simulink model "CCMVgaMotor"  (R2022b) (continued)
== system CCMVgaMotor == (continued)
  VentnActr04ElecErr_VentnActr04ElecErr/1 -> CCMVgaMotor_Runnable_sys/179(VentnActr04ElecErr_VentnActr04ElecErr_read)
  VentnActr04Mode_VentnActr04Mode/1 -> CCMVgaMotor_Runnable_sys/180(VentnActr04Mode_VentnActr04Mode_read)
  VentnActr04MovDir_VentnActr04MovDir/1 -> CCMVgaMotor_Runnable_sys/181(VentnActr04MovDir_VentnActr04MovDir_read)
  VentnActr04OvrTempErr_VentnActr04OvrTempErr/1 -> CCMVgaMotor_Runnable_sys/182(VentnActr04OvrTempErr_VentnActr04OvrTempErr_read)
  VentnActr04PsonSts_VentnActr04PsonSts/1 -> CCMVgaMotor_Runnable_sys/183(VentnActr04PsonSts_VentnActr04PsonSts_read)
  VentnActr04ResetSts_VentnActr04ResetSts/1 -> CCMVgaMotor_Runnable_sys/184(VentnActr04ResetSts_VentnActr04ResetSts_read)
  VentnActr04SpclFct_VentnActr04SpclFct/1 -> CCMVgaMotor_Runnable_sys/185(VentnActr04SpclFct_VentnActr04SpclFct_read)
  VentnActr04SpdSts_VentnActr04SpdSts/1 -> CCMVgaMotor_Runnable_sys/186(VentnActr04SpdSts_VentnActr04SpdSts_read)
  VentnActr04TrqSts_VentnActr04TrqSts/1 -> CCMVgaMotor_Runnable_sys/187(VentnActr04TrqSts_VentnActr04TrqSts_read)
  VentnActr04UrgcExist_VentnActr04UrgcExist/1 -> CCMVgaMotor_Runnable_sys/188(VentnActr04UrgcExist_VentnActr04UrgcExist_read)
  VentnActr04UrgcPson_VentnActr04UrgcPson/1 -> CCMVgaMotor_Runnable_sys/189(VentnActr04UrgcPson_VentnActr04UrgcPson_read)
  VentnActr04UrgcSts_VentnActr04UrgcSts/1 -> CCMVgaMotor_Runnable_sys/190(VentnActr04UrgcSts_VentnActr04UrgcSts_read)
  VentnActr04VoltErr_VentnActr04VoltErr/1 -> CCMVgaMotor_Runnable_sys/191(VentnActr04VoltErr_VentnActr04VoltErr_read)
  VentnActr05ActPson_VentnActr05ActPson/1 -> CCMVgaMotor_Runnable_sys/192(VentnActr05ActPson_VentnActr05ActPson_read)
  VentnActr05ActPson_VentnActr05ActPson_IsUpdated/1 -> CCMVgaMotor_Runnable_sys/193(VentnActr05ActPson_VentnActr05ActPson_IsUpdated_read)
  VentnActr05BlckInd_VentnActr05BlckInd/1 -> CCMVgaMotor_Runnable_sys/194(VentnActr05BlckInd_VentnActr05BlckInd_read)
  VentnActr05BlckSts_VentnActr05BlckSts/1 -> CCMVgaMotor_Runnable_sys/195(VentnActr05BlckSts_VentnActr05BlckSts_read)
  VentnActr05BusAdr_VentnActr05BusAdr/1 -> CCMVgaMotor_Runnable_sys/196(VentnActr05BusAdr_VentnActr05BusAdr_read)
  VentnActr05CoilSts_VentnActr05CoilSts/1 -> CCMVgaMotor_Runnable_sys/197(VentnActr05CoilSts_VentnActr05CoilSts_read)
  VentnActr05DrvDir_VentnActr05DrvDir/1 -> CCMVgaMotor_Runnable_sys/198(VentnActr05DrvDir_VentnActr05DrvDir_read)
  VentnActr05ElecErr_VentnActr05ElecErr/1 -> CCMVgaMotor_Runnable_sys/199(VentnActr05ElecErr_VentnActr05ElecErr_read)
  VentnActr05Mode_VentnActr05Mode/1 -> CCMVgaMotor_Runnable_sys/200(VentnActr05Mode_VentnActr05Mode_read)
  VentnActr05MovDir_VentnActr05MovDir/1 -> CCMVgaMotor_Runnable_sys/201(VentnActr05MovDir_VentnActr05MovDir_read)
  VentnActr05OvrTempErr_VentnActr05OvrTempErr/1 -> CCMVgaMotor_Runnable_sys/202(VentnActr05OvrTempErr_VentnActr05OvrTempErr_read)
  VentnActr05PsonSts_VentnActr05PsonSts/1 -> CCMVgaMotor_Runnable_sys/203(VentnActr05PsonSts_VentnActr05PsonSts_read)
  VentnActr05ResetSts_VentnActr05ResetSts/1 -> CCMVgaMotor_Runnable_sys/204(VentnActr05ResetSts_VentnActr05ResetSts_read)
  VentnActr05SpclFct_VentnActr05SpclFct/1 -> CCMVgaMotor_Runnable_sys/205(VentnActr05SpclFct_VentnActr05SpclFct_read)
  VentnActr05SpdSts_VentnActr05SpdSts/1 -> CCMVgaMotor_Runnable_sys/206(VentnActr05SpdSts_VentnActr05SpdSts_read)
  VentnActr05TrqSts_VentnActr05TrqSts/1 -> CCMVgaMotor_Runnable_sys/207(VentnActr05TrqSts_VentnActr05TrqSts_read)
  VentnActr05UrgcExist_VentnActr05UrgcExist/1 -> CCMVgaMotor_Runnable_sys/208(VentnActr05UrgcExist_VentnActr05UrgcExist_read)
  VentnActr05UrgcPson_VentnActr05UrgcPson/1 -> CCMVgaMotor_Runnable_sys/209(VentnActr05UrgcPson_VentnActr05UrgcPson_read)
  VentnActr05UrgcSts_VentnActr05UrgcSts/1 -> CCMVgaMotor_Runnable_sys/210(VentnActr05UrgcSts_VentnActr05UrgcSts_read)
  VentnActr05VoltErr_VentnActr05VoltErr/1 -> CCMVgaMotor_Runnable_sys/211(VentnActr05VoltErr_VentnActr05VoltErr_read)
  VentnActr06ActPson_VentnActr06ActPson/1 -> CCMVgaMotor_Runnable_sys/212(VentnActr06ActPson_VentnActr06ActPson_read)
  VentnActr06ActPson_VentnActr06ActPson_IsUpdated/1 -> CCMVgaMotor_Runnable_sys/213(VentnActr06ActPson_VentnActr06ActPson_IsUpdated_read)
  VentnActr06BlckInd_VentnActr06BlckInd/1 -> CCMVgaMotor_Runnable_sys/214(VentnActr06BlckInd_VentnActr06BlckInd_read)
  VentnActr06BlckSts_VentnActr06BlckSts/1 -> CCMVgaMotor_Runnable_sys/215(VentnActr06BlckSts_VentnActr06BlckSts_read)
  VentnActr06BusAdr_VentnActr06BusAdr/1 -> CCMVgaMotor_Runnable_sys/216(VentnActr06BusAdr_VentnActr06BusAdr_read)
  VentnActr06CoilSts_VentnActr06CoilSts/1 -> CCMVgaMotor_Runnable_sys/217(VentnActr06CoilSts_VentnActr06CoilSts_read)
  VentnActr06DrvDir_VentnActr06DrvDir/1 -> CCMVgaMotor_Runnable_sys/218(VentnActr06DrvDir_VentnActr06DrvDir_read)
  VentnActr06ElecErr_VentnActr06ElecErr/1 -> CCMVgaMotor_Runnable_sys/219(VentnActr06ElecErr_VentnActr06ElecErr_read)
  VentnActr06Mode_VentnActr06Mode/1 -> CCMVgaMotor_Runnable_sys/220(VentnActr06Mode_VentnActr06Mode_read)
  VentnActr06MovDir_VentnActr06MovDir/1 -> CCMVgaMotor_Runnable_sys/221(VentnActr06MovDir_VentnActr06MovDir_read)
  VentnActr06OvrTempErr_VentnActr06OvrTempErr/1 -> CCMVgaMotor_Runnable_sys/222(VentnActr06OvrTempErr_VentnActr06OvrTempErr_read)
  VentnActr06PsonSts_VentnActr06PsonSts/1 -> CCMVgaMotor_Runnable_sys/223(VentnActr06PsonSts_VentnActr06PsonSts_read)
  VentnActr06ResetSts_VentnActr06ResetSts/1 -> CCMVgaMotor_Runnable_sys/224(VentnActr06ResetSts_VentnActr06ResetSts_read)
  VentnActr06SpclFct_VentnActr06SpclFct/1 -> CCMVgaMotor_Runnable_sys/225(VentnActr06SpclFct_VentnActr06SpclFct_read)
  VentnActr06SpdSts_VentnActr06SpdSts/1 -> CCMVgaMotor_Runnable_sys/226(VentnActr06SpdSts_VentnActr06SpdSts_read)
  VentnActr06TrqSts_VentnActr06TrqSts/1 -> CCMVgaMotor_Runnable_sys/227(VentnActr06TrqSts_VentnActr06TrqSts_read)
  VentnActr06UrgcExist_VentnActr06UrgcExist/1 -> CCMVgaMotor_Runnable_sys/228(VentnActr06UrgcExist_VentnActr06UrgcExist_read)
  VentnActr06UrgcPson_VentnActr06UrgcPson/1 -> CCMVgaMotor_Runnable_sys/229(VentnActr06UrgcPson_VentnActr06UrgcPson_read)
  VentnActr06UrgcSts_VentnActr06UrgcSts/1 -> CCMVgaMotor_Runnable_sys/230(VentnActr06UrgcSts_VentnActr06UrgcSts_read)
  VentnActr06VoltErr_VentnActr06VoltErr/1 -> CCMVgaMotor_Runnable_sys/231(VentnActr06VoltErr_VentnActr06VoltErr_read)
  VentnActr07ActPson_VentnActr07ActPson/1 -> CCMVgaMotor_Runnable_sys/232(VentnActr07ActPson_VentnActr07ActPson_read)
  VentnActr07ActPson_VentnActr07ActPson_IsUpdated/1 -> CCMVgaMotor_Runnable_sys/233(VentnActr07ActPson_VentnActr07ActPson_IsUpdated_read)
  VentnActr07BlckInd_VentnActr07BlckInd/1 -> CCMVgaMotor_Runnable_sys/234(VentnActr07BlckInd_VentnActr07BlckInd_read)
  VentnActr07BlckSts_VentnActr07BlckSts/1 -> CCMVgaMotor_Runnable_sys/235(VentnActr07BlckSts_VentnActr07BlckSts_read)
  VentnActr07BusAdr_VentnActr07BusAdr/1 -> CCMVgaMotor_Runnable_sys/236(VentnActr07BusAdr_VentnActr07BusAdr_read)
  VentnActr07CoilSts_VentnActr07CoilSts/1 -> CCMVgaMotor_Runnable_sys/237(VentnActr07CoilSts_VentnActr07CoilSts_read)
  VentnActr07DrvDir_VentnActr07DrvDir/1 -> CCMVgaMotor_Runnable_sys/238(VentnActr07DrvDir_VentnActr07DrvDir_read)
  VentnActr07ElecErr_VentnActr07ElecErr/1 -> CCMVgaMotor_Runnable_sys/239(VentnActr07ElecErr_VentnActr07ElecErr_read)
  VentnActr07Mode_VentnActr07Mode/1 -> CCMVgaMotor_Runnable_sys/240(VentnActr07Mode_VentnActr07Mode_read)
  VentnActr07MovDir_VentnActr07MovDir/1 -> CCMVgaMotor_Runnable_sys/241(VentnActr07MovDir_VentnActr07MovDir_read)
  VentnActr07OvrTempErr_VentnActr07OvrTempErr/1 -> CCMVgaMotor_Runnable_sys/242(VentnActr07OvrTempErr_VentnActr07OvrTempErr_read)
  VentnActr07PsonSts_VentnActr07PsonSts/1 -> CCMVgaMotor_Runnable_sys/243(VentnActr07PsonSts_VentnActr07PsonSts_read)
  VentnActr07ResetSts_VentnActr07ResetSts/1 -> CCMVgaMotor_Runnable_sys/244(VentnActr07ResetSts_VentnActr07ResetSts_read)
  VentnActr07SpclFct_VentnActr07SpclFct/1 -> CCMVgaMotor_Runnable_sys/245(VentnActr07SpclFct_VentnActr07SpclFct_read)
  VentnActr07SpdSts_VentnActr07SpdSts/1 -> CCMVgaMotor_Runnable_sys/246(VentnActr07SpdSts_VentnActr07SpdSts_read)
  VentnActr07TrqSts_VentnActr07TrqSts/1 -> CCMVgaMotor_Runnable_sys/247(VentnActr07TrqSts_VentnActr07TrqSts_read)
  VentnActr07UrgcExist_VentnActr07UrgcExist/1 -> CCMVgaMotor_Runnable_sys/248(VentnActr07UrgcExist_VentnActr07UrgcExist_read)
  VentnActr07UrgcPson_VentnActr07UrgcPson/1 -> CCMVgaMotor_Runnable_sys/249(VentnActr07UrgcPson_VentnActr07UrgcPson_read)
  VentnActr07UrgcSts_VentnActr07UrgcSts/1 -> CCMVgaMotor_Runnable_sys/250(VentnActr07UrgcSts_VentnActr07UrgcSts_read)
  VentnActr07VoltErr_VentnActr07VoltErr/1 -> CCMVgaMotor_Runnable_sys/251(VentnActr07VoltErr_VentnActr07VoltErr_read)
  VentnActr08ActPson_VentnActr08ActPson/1 -> CCMVgaMotor_Runnable_sys/252(VentnActr08ActPson_VentnActr08ActPson_read)
  VentnActr08ActPson_VentnActr08ActPson_IsUpdated/1 -> CCMVgaMotor_Runnable_sys/253(VentnActr08ActPson_VentnActr08ActPson_IsUpdated_read)
  VentnActr08BlckInd_VentnActr08BlckInd/1 -> CCMVgaMotor_Runnable_sys/254(VentnActr08BlckInd_VentnActr08BlckInd_read)
  VentnActr08BlckSts_VentnActr08BlckSts/1 -> CCMVgaMotor_Runnable_sys/255(VentnActr08BlckSts_VentnActr08BlckSts_read)
  VentnActr08BusAdr_VentnActr08BusAdr/1 -> CCMVgaMotor_Runnable_sys/256(VentnActr08BusAdr_VentnActr08BusAdr_read)
  VentnActr08CoilSts_VentnActr08CoilSts/1 -> CCMVgaMotor_Runnable_sys/257(VentnActr08CoilSts_VentnActr08CoilSts_read)
  VentnActr08DrvDir_VentnActr08DrvDir/1 -> CCMVgaMotor_Runnable_sys/258(VentnActr08DrvDir_VentnActr08DrvDir_read)
  VentnActr08ElecErr_VentnActr08ElecErr/1 -> CCMVgaMotor_Runnable_sys/259(VentnActr08ElecErr_VentnActr08ElecErr_read)
  VentnActr08Mode_VentnActr08Mode/1 -> CCMVgaMotor_Runnable_sys/260(VentnActr08Mode_VentnActr08Mode_read)
  VentnActr08MovDir_VentnActr08MovDir/1 -> CCMVgaMotor_Runnable_sys/261(VentnActr08MovDir_VentnActr08MovDir_read)
  VentnActr08OvrTempErr_VentnActr08OvrTempErr/1 -> CCMVgaMotor_Runnable_sys/262(VentnActr08OvrTempErr_VentnActr08OvrTempErr_read)
  VentnActr08PsonSts_VentnActr08PsonSts/1 -> CCMVgaMotor_Runnable_sys/263(VentnActr08PsonSts_VentnActr08PsonSts_read)
  VentnActr08ResetSts_VentnActr08ResetSts/1 -> CCMVgaMotor_Runnable_sys/264(VentnActr08ResetSts_VentnActr08ResetSts_read)
  VentnActr08SpclFct_VentnActr08SpclFct/1 -> CCMVgaMotor_Runnable_sys/265(VentnActr08SpclFct_VentnActr08SpclFct_read)
  VentnActr08SpdSts_VentnActr08SpdSts/1 -> CCMVgaMotor_Runnable_sys/266(VentnActr08SpdSts_VentnActr08SpdSts_read)
  VentnActr08TrqSts_VentnActr08TrqSts/1 -> CCMVgaMotor_Runnable_sys/267(VentnActr08TrqSts_VentnActr08TrqSts_read)
  VentnActr08UrgcExist_VentnActr08UrgcExist/1 -> CCMVgaMotor_Runnable_sys/268(VentnActr08UrgcExist_VentnActr08UrgcExist_read)
  VentnActr08UrgcPson_VentnActr08UrgcPson/1 -> CCMVgaMotor_Runnable_sys/269(VentnActr08UrgcPson_VentnActr08UrgcPson_read)
  VentnActr08UrgcSts_VentnActr08UrgcSts/1 -> CCMVgaMotor_Runnable_sys/270(VentnActr08UrgcSts_VentnActr08UrgcSts_read)
  VentnActr08VoltErr_VentnActr08VoltErr/1 -> CCMVgaMotor_Runnable_sys/271(VentnActr08VoltErr_VentnActr08VoltErr_read)
  VentnActr09ActPson_VentnActr09ActPson/1 -> CCMVgaMotor_Runnable_sys/272(VentnActr09ActPson_VentnActr09ActPson_read)
  VentnActr09ActPson_VentnActr09ActPson_IsUpdated/1 -> CCMVgaMotor_Runnable_sys/273(VentnActr09ActPson_VentnActr09ActPson_IsUpdated_read)
  VentnActr09BlckInd_VentnActr09BlckInd/1 -> CCMVgaMotor_Runnable_sys/274(VentnActr09BlckInd_VentnActr09BlckInd_read)
  VentnActr09BlckSts_VentnActr09BlckSts/1 -> CCMVgaMotor_Runnable_sys/275(VentnActr09BlckSts_VentnActr09BlckSts_read)
  VentnActr09BusAdr_VentnActr09BusAdr/1 -> CCMVgaMotor_Runnable_sys/276(VentnActr09BusAdr_VentnActr09BusAdr_read)
  VentnActr09CoilSts_VentnActr09CoilSts/1 -> CCMVgaMotor_Runnable_sys/277(VentnActr09CoilSts_VentnActr09CoilSts_read)
  VentnActr09DrvDir_VentnActr09DrvDir/1 -> CCMVgaMotor_Runnable_sys/278(VentnActr09DrvDir_VentnActr09DrvDir_read)
  VentnActr09ElecErr_VentnActr09ElecErr/1 -> CCMVgaMotor_Runnable_sys/279(VentnActr09ElecErr_VentnActr09ElecErr_read)
  VentnActr09Mode_VentnActr09Mode/1 -> CCMVgaMotor_Runnable_sys/280(VentnActr09Mode_VentnActr09Mode_read)
  VentnActr09MovDir_VentnActr09MovDir/1 -> CCMVgaMotor_Runnable_sys/281(VentnActr09MovDir_VentnActr09MovDir_read)
  VentnActr09OvrTempErr_VentnActr09OvrTempErr/1 -> CCMVgaMotor_Runnable_sys/282(VentnActr09OvrTempErr_VentnActr09OvrTempErr_read)
  VentnActr09PsonSts_VentnActr09PsonSts/1 -> CCMVgaMotor_Runnable_sys/283(VentnActr09PsonSts_VentnActr09PsonSts_read)
  VentnActr09ResetSts_VentnActr09ResetSts/1 -> CCMVgaMotor_Runnable_sys/284(VentnActr09ResetSts_VentnActr09ResetSts_read)
  VentnActr09SpclFct_VentnActr09SpclFct/1 -> CCMVgaMotor_Runnable_sys/285(VentnActr09SpclFct_VentnActr09SpclFct_read)
  VentnActr09SpdSts_VentnActr09SpdSts/1 -> CCMVgaMotor_Runnable_sys/286(VentnActr09SpdSts_VentnActr09SpdSts_read)
  VentnActr09TrqSts_VentnActr09TrqSts/1 -> CCMVgaMotor_Runnable_sys/287(VentnActr09TrqSts_VentnActr09TrqSts_read)
  VentnActr09UrgcExist_VentnActr09UrgcExist/1 -> CCMVgaMotor_Runnable_sys/288(VentnActr09UrgcExist_VentnActr09UrgcExist_read)
  VentnActr09UrgcPson_VentnActr09UrgcPson/1 -> CCMVgaMotor_Runnable_sys/289(VentnActr09UrgcPson_VentnActr09UrgcPson_read)
  VentnActr09UrgcSts_VentnActr09UrgcSts/1 -> CCMVgaMotor_Runnable_sys/290(VentnActr09UrgcSts_VentnActr09UrgcSts_read)
  VentnActr09VoltErr_VentnActr09VoltErr/1 -> CCMVgaMotor_Runnable_sys/291(VentnActr09VoltErr_VentnActr09VoltErr_read)
  VentnActr10ActPson_VentnActr10ActPson/1 -> CCMVgaMotor_Runnable_sys/292(VentnActr10ActPson_VentnActr10ActPson_read)
  VentnActr10ActPson_VentnActr10ActPson_IsUpdated/1 -> CCMVgaMotor_Runnable_sys/293(VentnActr10ActPson_VentnActr10ActPson_IsUpdated_read)
  VentnActr10BlckInd_VentnActr10BlckInd/1 -> CCMVgaMotor_Runnable_sys/294(VentnActr10BlckInd_VentnActr10BlckInd_read)
  VentnActr10BlckSts_VentnActr10BlckSts/1 -> CCMVgaMotor_Runnable_sys/295(VentnActr10BlckSts_VentnActr10BlckSts_read)
  VentnActr10BusAdr_VentnActr10BusAdr/1 -> CCMVgaMotor_Runnable_sys/296(VentnActr10BusAdr_VentnActr10BusAdr_read)
  VentnActr10CoilSts_VentnActr10CoilSts/1 -> CCMVgaMotor_Runnable_sys/297(VentnActr10CoilSts_VentnActr10CoilSts_read)
  VentnActr10DrvDir_VentnActr10DrvDir/1 -> CCMVgaMotor_Runnable_sys/298(VentnActr10DrvDir_VentnActr10DrvDir_read)
  VentnActr10ElecErr_VentnActr10ElecErr/1 -> CCMVgaMotor_Runnable_sys/299(VentnActr10ElecErr_VentnActr10ElecErr_read)
  VentnActr10Mode_VentnActr10Mode/1 -> CCMVgaMotor_Runnable_sys/300(VentnActr10Mode_VentnActr10Mode_read)
  VentnActr10MovDir_VentnActr10MovDir/1 -> CCMVgaMotor_Runnable_sys/301(VentnActr10MovDir_VentnActr10MovDir_read)
  VentnActr10OvrTempErr_VentnActr10OvrTempErr/1 -> CCMVgaMotor_Runnable_sys/302(VentnActr10OvrTempErr_VentnActr10OvrTempErr_read)
  VentnActr10PsonSts_VentnActr10PsonSts/1 -> CCMVgaMotor_Runnable_sys/303(VentnActr10PsonSts_VentnActr10PsonSts_read)
  VentnActr10ResetSts_VentnActr10ResetSts/1 -> CCMVgaMotor_Runnable_sys/304(VentnActr10ResetSts_VentnActr10ResetSts_read)
  VentnActr10SpclFct_VentnActr10SpclFct/1 -> CCMVgaMotor_Runnable_sys/305(VentnActr10SpclFct_VentnActr10SpclFct_read)
  VentnActr10SpdSts_VentnActr10SpdSts/1 -> CCMVgaMotor_Runnable_sys/306(VentnActr10SpdSts_VentnActr10SpdSts_read)
  VentnActr10TrqSts_VentnActr10TrqSts/1 -> CCMVgaMotor_Runnable_sys/307(VentnActr10TrqSts_VentnActr10TrqSts_read)
  VentnActr10UrgcExist_VentnActr10UrgcExist/1 -> CCMVgaMotor_Runnable_sys/308(VentnActr10UrgcExist_VentnActr10UrgcExist_read)
  VentnActr10UrgcPson_VentnActr10UrgcPson/1 -> CCMVgaMotor_Runnable_sys/309(VentnActr10UrgcPson_VentnActr10UrgcPson_read)
  VentnActr10UrgcSts_VentnActr10UrgcSts/1 -> CCMVgaMotor_Runnable_sys/310(VentnActr10UrgcSts_VentnActr10UrgcSts_read)
  VentnActr10VoltErr_VentnActr10VoltErr/1 -> CCMVgaMotor_Runnable_sys/311(VentnActr10VoltErr_VentnActr10VoltErr_read)
  VentnActr11ActPson_VentnActr11ActPson/1 -> CCMVgaMotor_Runnable_sys/312(VentnActr11ActPson_VentnActr11ActPson_read)
  VentnActr11ActPson_VentnActr11ActPson_IsUpdated/1 -> CCMVgaMotor_Runnable_sys/313(VentnActr11ActPson_VentnActr11ActPson_IsUpdated_read)
  VentnActr11BlckInd_VentnActr11BlckInd/1 -> CCMVgaMotor_Runnable_sys/314(VentnActr11BlckInd_VentnActr11BlckInd_read)
  VentnActr11BlckSts_VentnActr11BlckSts/1 -> CCMVgaMotor_Runnable_sys/315(VentnActr11BlckSts_VentnActr11BlckSts_read)
  VentnActr11BusAdr_VentnActr11BusAdr/1 -> CCMVgaMotor_Runnable_sys/316(VentnActr11BusAdr_VentnActr11BusAdr_read)
  VentnActr11CoilSts_VentnActr11CoilSts/1 -> CCMVgaMotor_Runnable_sys/317(VentnActr11CoilSts_VentnActr11CoilSts_read)
  VentnActr11DrvDir_VentnActr11DrvDir/1 -> CCMVgaMotor_Runnable_sys/318(VentnActr11DrvDir_VentnActr11DrvDir_read)
  VentnActr11ElecErr_VentnActr11ElecErr/1 -> CCMVgaMotor_Runnable_sys/319(VentnActr11ElecErr_VentnActr11ElecErr_read)
  VentnActr11Mode_VentnActr11Mode/1 -> CCMVgaMotor_Runnable_sys/320(VentnActr11Mode_VentnActr11Mode_read)
  VentnActr11MovDir_VentnActr11MovDir/1 -> CCMVgaMotor_Runnable_sys/321(VentnActr11MovDir_VentnActr11MovDir_read)
  VentnActr11OvrTempErr_VentnActr11OvrTempErr/1 -> CCMVgaMotor_Runnable_sys/322(VentnActr11OvrTempErr_VentnActr11OvrTempErr_read)
  VentnActr11PsonSts_VentnActr11PsonSts/1 -> CCMVgaMotor_Runnable_sys/323(VentnActr11PsonSts_VentnActr11PsonSts_read)
  VentnActr11ResetSts_VentnActr11ResetSts/1 -> CCMVgaMotor_Runnable_sys/324(VentnActr11ResetSts_VentnActr11ResetSts_read)
  VentnActr11SpclFct_VentnActr11SpclFct/1 -> CCMVgaMotor_Runnable_sys/325(VentnActr11SpclFct_VentnActr11SpclFct_read)
  VentnActr11SpdSts_VentnActr11SpdSts/1 -> CCMVgaMotor_Runnable_sys/326(VentnActr11SpdSts_VentnActr11SpdSts_read)
  VentnActr11TrqSts_VentnActr11TrqSts/1 -> CCMVgaMotor_Runnable_sys/327(VentnActr11TrqSts_VentnActr11TrqSts_read)
  VentnActr11UrgcExist_VentnActr11UrgcExist/1 -> CCMVgaMotor_Runnable_sys/328(VentnActr11UrgcExist_VentnActr11UrgcExist_read)
  VentnActr11UrgcPson_VentnActr11UrgcPson/1 -> CCMVgaMotor_Runnable_sys/329(VentnActr11UrgcPson_VentnActr11UrgcPson_read)
  VentnActr11UrgcSts_VentnActr11UrgcSts/1 -> CCMVgaMotor_Runnable_sys/330(VentnActr11UrgcSts_VentnActr11UrgcSts_read)
  VentnActr11VoltErr_VentnActr11VoltErr/1 -> CCMVgaMotor_Runnable_sys/331(VentnActr11VoltErr_VentnActr11VoltErr_read)
  VentnActr12ActPson_VentnActr12ActPson/1 -> CCMVgaMotor_Runnable_sys/332(VentnActr12ActPson_VentnActr12ActPson_read)
  VentnActr12ActPson_VentnActr12ActPson_IsUpdated/1 -> CCMVgaMotor_Runnable_sys/333(VentnActr12ActPson_VentnActr12ActPson_IsUpdated_read)
  VentnActr12BlckInd_VentnActr12BlckInd/1 -> CCMVgaMotor_Runnable_sys/334(VentnActr12BlckInd_VentnActr12BlckInd_read)
  VentnActr12BlckSts_VentnActr12BlckSts/1 -> CCMVgaMotor_Runnable_sys/335(VentnActr12BlckSts_VentnActr12BlckSts_read)
  VentnActr12BusAdr_VentnActr12BusAdr/1 -> CCMVgaMotor_Runnable_sys/336(VentnActr12BusAdr_VentnActr12BusAdr_read)
  VentnActr12CoilSts_VentnActr12CoilSts/1 -> CCMVgaMotor_Runnable_sys/337(VentnActr12CoilSts_VentnActr12CoilSts_read)
  VentnActr12DrvDir_VentnActr12DrvDir/1 -> CCMVgaMotor_Runnable_sys/338(VentnActr12DrvDir_VentnActr12DrvDir_read)
  VentnActr12ElecErr_VentnActr12ElecErr/1 -> CCMVgaMotor_Runnable_sys/339(VentnActr12ElecErr_VentnActr12ElecErr_read)
  VentnActr12Mode_VentnActr12Mode/1 -> CCMVgaMotor_Runnable_sys/340(VentnActr12Mode_VentnActr12Mode_read)
  VentnActr12MovDir_VentnActr12MovDir/1 -> CCMVgaMotor_Runnable_sys/341(VentnActr12MovDir_VentnActr12MovDir_read)
  VentnActr12OvrTempErr_VentnActr12OvrTempErr/1 -> CCMVgaMotor_Runnable_sys/342(VentnActr12OvrTempErr_VentnActr12OvrTempErr_read)
  VentnActr12PsonSts_VentnActr12PsonSts/1 -> CCMVgaMotor_Runnable_sys/343(VentnActr12PsonSts_VentnActr12PsonSts_read)
  VentnActr12ResetSts_VentnActr12ResetSts/1 -> CCMVgaMotor_Runnable_sys/344(VentnActr12ResetSts_VentnActr12ResetSts_read)
  VentnActr12SpclFct_VentnActr12SpclFct/1 -> CCMVgaMotor_Runnable_sys/345(VentnActr12SpclFct_VentnActr12SpclFct_read)
  VentnActr12SpdSts_VentnActr12SpdSts/1 -> CCMVgaMotor_Runnable_sys/346(VentnActr12SpdSts_VentnActr12SpdSts_read)
  VentnActr12TrqSts_VentnActr12TrqSts/1 -> CCMVgaMotor_Runnable_sys/347(VentnActr12TrqSts_VentnActr12TrqSts_read)
  VentnActr12UrgcExist_VentnActr12UrgcExist/1 -> CCMVgaMotor_Runnable_sys/348(VentnActr12UrgcExist_VentnActr12UrgcExist_read)
  VentnActr12UrgcPson_VentnActr12UrgcPson/1 -> CCMVgaMotor_Runnable_sys/349(VentnActr12UrgcPson_VentnActr12UrgcPson_read)
  VentnActr12UrgcSts_VentnActr12UrgcSts/1 -> CCMVgaMotor_Runnable_sys/350(VentnActr12UrgcSts_VentnActr12UrgcSts_read)
  VentnActr12VoltErr_VentnActr12VoltErr/1 -> CCMVgaMotor_Runnable_sys/351(VentnActr12VoltErr_VentnActr12VoltErr_read)
  VentnActr13ActPson_VentnActr13ActPson/1 -> CCMVgaMotor_Runnable_sys/352(VentnActr13ActPson_VentnActr13ActPson_read)
  VentnActr13ActPson_VentnActr13ActPson_IsUpdated/1 -> CCMVgaMotor_Runnable_sys/353(VentnActr13ActPson_VentnActr13ActPson_IsUpdated_read)
  VentnActr13BlckInd_VentnActr13BlckInd/1 -> CCMVgaMotor_Runnable_sys/354(VentnActr13BlckInd_VentnActr13BlckInd_read)
  VentnActr13BlckSts_VentnActr13BlckSts/1 -> CCMVgaMotor_Runnable_sys/355(VentnActr13BlckSts_VentnActr13BlckSts_read)
  VentnActr13BusAdr_VentnActr13BusAdr/1 -> CCMVgaMotor_Runnable_sys/356(VentnActr13BusAdr_VentnActr13BusAdr_read)
  VentnActr13CoilSts_VentnActr13CoilSts/1 -> CCMVgaMotor_Runnable_sys/357(VentnActr13CoilSts_VentnActr13CoilSts_read)
  VentnActr13DrvDir_VentnActr13DrvDir/1 -> CCMVgaMotor_Runnable_sys/358(VentnActr13DrvDir_VentnActr13DrvDir_read)
  VentnActr13ElecErr_VentnActr13ElecErr/1 -> CCMVgaMotor_Runnable_sys/359(VentnActr13ElecErr_VentnActr13ElecErr_read)
  VentnActr13Mode_VentnActr13Mode/1 -> CCMVgaMotor_Runnable_sys/360(VentnActr13Mode_VentnActr13Mode_read)
  VentnActr13MovDir_VentnActr13MovDir/1 -> CCMVgaMotor_Runnable_sys/361(VentnActr13MovDir_VentnActr13MovDir_read)
  VentnActr13OvrTempErr_VentnActr13OvrTempErr/1 -> CCMVgaMotor_Runnable_sys/362(VentnActr13OvrTempErr_VentnActr13OvrTempErr_read)
  VentnActr13PsonSts_VentnActr13PsonSts/1 -> CCMVgaMotor_Runnable_sys/363(VentnActr13PsonSts_VentnActr13PsonSts_read)
  VentnActr13ResetSts_VentnActr13ResetSts/1 -> CCMVgaMotor_Runnable_sys/364(VentnActr13ResetSts_VentnActr13ResetSts_read)
  VentnActr13SpclFct_VentnActr13SpclFct/1 -> CCMVgaMotor_Runnable_sys/365(VentnActr13SpclFct_VentnActr13SpclFct_read)
  VentnActr13SpdSts_VentnActr13SpdSts/1 -> CCMVgaMotor_Runnable_sys/366(VentnActr13SpdSts_VentnActr13SpdSts_read)
  VentnActr13TrqSts_VentnActr13TrqSts/1 -> CCMVgaMotor_Runnable_sys/367(VentnActr13TrqSts_VentnActr13TrqSts_read)
  VentnActr13UrgcExist_VentnActr13UrgcExist/1 -> CCMVgaMotor_Runnable_sys/368(VentnActr13UrgcExist_VentnActr13UrgcExist_read)
  VentnActr13UrgcPson_VentnActr13UrgcPson/1 -> CCMVgaMotor_Runnable_sys/369(VentnActr13UrgcPson_VentnActr13UrgcPson_read)
  VentnActr13UrgcSts_VentnActr13UrgcSts/1 -> CCMVgaMotor_Runnable_sys/370(VentnActr13UrgcSts_VentnActr13UrgcSts_read)
  VentnActr13VoltErr_VentnActr13VoltErr/1 -> CCMVgaMotor_Runnable_sys/371(VentnActr13VoltErr_VentnActr13VoltErr_read)
  VentnActr14ActPson_VentnActr14ActPson/1 -> CCMVgaMotor_Runnable_sys/372(VentnActr14ActPson_VentnActr14ActPson_read)
  VentnActr14ActPson_VentnActr14ActPson_IsUpdated/1 -> CCMVgaMotor_Runnable_sys/373(VentnActr14ActPson_VentnActr14ActPson_IsUpdated_read)
  VentnActr14BlckInd_VentnActr14BlckInd/1 -> CCMVgaMotor_Runnable_sys/374(VentnActr14BlckInd_VentnActr14BlckInd_read)
  VentnActr14BlckSts_VentnActr14BlckSts/1 -> CCMVgaMotor_Runnable_sys/375(VentnActr14BlckSts_VentnActr14BlckSts_read)
  VentnActr14BusAdr_VentnActr14BusAdr/1 -> CCMVgaMotor_Runnable_sys/376(VentnActr14BusAdr_VentnActr14BusAdr_read)
  VentnActr14CoilSts_VentnActr14CoilSts/1 -> CCMVgaMotor_Runnable_sys/377(VentnActr14CoilSts_VentnActr14CoilSts_read)
  VentnActr14DrvDir_VentnActr14DrvDir/1 -> CCMVgaMotor_Runnable_sys/378(VentnActr14DrvDir_VentnActr14DrvDir_read)
  VentnActr14ElecErr_VentnActr14ElecErr/1 -> CCMVgaMotor_Runnable_sys/379(VentnActr14ElecErr_VentnActr14ElecErr_read)
  VentnActr14Mode_VentnActr14Mode/1 -> CCMVgaMotor_Runnable_sys/380(VentnActr14Mode_VentnActr14Mode_read)
  VentnActr14MovDir_VentnActr14MovDir/1 -> CCMVgaMotor_Runnable_sys/381(VentnActr14MovDir_VentnActr14MovDir_read)
  VentnActr14OvrTempErr_VentnActr14OvrTempErr/1 -> CCMVgaMotor_Runnable_sys/382(VentnActr14OvrTempErr_VentnActr14OvrTempErr_read)
  VentnActr14PsonSts_VentnActr14PsonSts/1 -> CCMVgaMotor_Runnable_sys/383(VentnActr14PsonSts_VentnActr14PsonSts_read)
  VentnActr14ResetSts_VentnActr14ResetSts/1 -> CCMVgaMotor_Runnable_sys/384(VentnActr14ResetSts_VentnActr14ResetSts_read)
  VentnActr14SpclFct_VentnActr14SpclFct/1 -> CCMVgaMotor_Runnable_sys/385(VentnActr14SpclFct_VentnActr14SpclFct_read)
  VentnActr14SpdSts_VentnActr14SpdSts/1 -> CCMVgaMotor_Runnable_sys/386(VentnActr14SpdSts_VentnActr14SpdSts_read)
  VentnActr14TrqSts_VentnActr14TrqSts/1 -> CCMVgaMotor_Runnable_sys/387(VentnActr14TrqSts_VentnActr14TrqSts_read)
  VentnActr14UrgcExist_VentnActr14UrgcExist/1 -> CCMVgaMotor_Runnable_sys/388(VentnActr14UrgcExist_VentnActr14UrgcExist_read)
  VentnActr14UrgcPson_VentnActr14UrgcPson/1 -> CCMVgaMotor_Runnable_sys/389(VentnActr14UrgcPson_VentnActr14UrgcPson_read)
  VentnActr14UrgcSts_VentnActr14UrgcSts/1 -> CCMVgaMotor_Runnable_sys/390(VentnActr14UrgcSts_VentnActr14UrgcSts_read)
  VentnActr14VoltErr_VentnActr14VoltErr/1 -> CCMVgaMotor_Runnable_sys/391(VentnActr14VoltErr_VentnActr14VoltErr_read)
  VentnActr15ActPson_VentnActr15ActPson/1 -> CCMVgaMotor_Runnable_sys/392(VentnActr15ActPson_VentnActr15ActPson_read)
  VentnActr15ActPson_VentnActr15ActPson_IsUpdated/1 -> CCMVgaMotor_Runnable_sys/393(VentnActr15ActPson_VentnActr15ActPson_IsUpdated_read)
  VentnActr15BlckInd_VentnActr15BlckInd/1 -> CCMVgaMotor_Runnable_sys/394(VentnActr15BlckInd_VentnActr15BlckInd_read)
  VentnActr15BlckSts_VentnActr15BlckSts/1 -> CCMVgaMotor_Runnable_sys/395(VentnActr15BlckSts_VentnActr15BlckSts_read)
  VentnActr15BusAdr_VentnActr15BusAdr/1 -> CCMVgaMotor_Runnable_sys/396(VentnActr15BusAdr_VentnActr15BusAdr_read)
  VentnActr15CoilSts_VentnActr15CoilSts/1 -> CCMVgaMotor_Runnable_sys/397(VentnActr15CoilSts_VentnActr15CoilSts_read)
  VentnActr15DrvDir_VentnActr15DrvDir/1 -> CCMVgaMotor_Runnable_sys/398(VentnActr15DrvDir_VentnActr15DrvDir_read)
  VentnActr15ElecErr_VentnActr15ElecErr/1 -> CCMVgaMotor_Runnable_sys/399(VentnActr15ElecErr_VentnActr15ElecErr_read)
  VentnActr15Mode_VentnActr15Mode/1 -> CCMVgaMotor_Runnable_sys/400(VentnActr15Mode_VentnActr15Mode_read)
  VentnActr15MovDir_VentnActr15MovDir/1 -> CCMVgaMotor_Runnable_sys/401(VentnActr15MovDir_VentnActr15MovDir_read)
  VentnActr15OvrTempErr_VentnActr15OvrTempErr/1 -> CCMVgaMotor_Runnable_sys/402(VentnActr15OvrTempErr_VentnActr15OvrTempErr_read)
  VentnActr15PsonSts_VentnActr15PsonSts/1 -> CCMVgaMotor_Runnable_sys/403(VentnActr15PsonSts_VentnActr15PsonSts_read)
  VentnActr15ResetSts_VentnActr15ResetSts/1 -> CCMVgaMotor_Runnable_sys/404(VentnActr15ResetSts_VentnActr15ResetSts_read)
  VentnActr15SpclFct_VentnActr15SpclFct/1 -> CCMVgaMotor_Runnable_sys/405(VentnActr15SpclFct_VentnActr15SpclFct_read)
  VentnActr15SpdSts_VentnActr15SpdSts/1 -> CCMVgaMotor_Runnable_sys/406(VentnActr15SpdSts_VentnActr15SpdSts_read)
  VentnActr15TrqSts_VentnActr15TrqSts/1 -> CCMVgaMotor_Runnable_sys/407(VentnActr15TrqSts_VentnActr15TrqSts_read)
  VentnActr15UrgcExist_VentnActr15UrgcExist/1 -> CCMVgaMotor_Runnable_sys/408(VentnActr15UrgcExist_VentnActr15UrgcExist_read)
  VentnActr15UrgcPson_VentnActr15UrgcPson/1 -> CCMVgaMotor_Runnable_sys/409(VentnActr15UrgcPson_VentnActr15UrgcPson_read)
  VentnActr15UrgcSts_VentnActr15UrgcSts/1 -> CCMVgaMotor_Runnable_sys/410(VentnActr15UrgcSts_VentnActr15UrgcSts_read)
  VentnActr15VoltErr_VentnActr15VoltErr/1 -> CCMVgaMotor_Runnable_sys/411(VentnActr15VoltErr_VentnActr15VoltErr_read)
  VentnActr16ActPson_VentnActr16ActPson/1 -> CCMVgaMotor_Runnable_sys/412(VentnActr16ActPson_VentnActr16ActPson_read)
  VentnActr16BlckInd_VentnActr16BlckInd/1 -> CCMVgaMotor_Runnable_sys/413(VentnActr16BlckInd_VentnActr16BlckInd_read)
  VentnActr16BlckSts_VentnActr16BlckSts/1 -> CCMVgaMotor_Runnable_sys/414(VentnActr16BlckSts_VentnActr16BlckSts_read)
  VentnActr16BusAdr_VentnActr16BusAdr/1 -> CCMVgaMotor_Runnable_sys/415(VentnActr16BusAdr_VentnActr16BusAdr_read)
  VentnActr16CoilSts_VentnActr16CoilSts/1 -> CCMVgaMotor_Runnable_sys/416(VentnActr16CoilSts_VentnActr16CoilSts_read)
  VentnActr16DrvDir_VentnActr16DrvDir/1 -> CCMVgaMotor_Runnable_sys/417(VentnActr16DrvDir_VentnActr16DrvDir_read)
  VentnActr16ElecErr_VentnActr16ElecErr/1 -> CCMVgaMotor_Runnable_sys/418(VentnActr16ElecErr_VentnActr16ElecErr_read)
  VentnActr16Mode_VentnActr16Mode/1 -> CCMVgaMotor_Runnable_sys/419(VentnActr16Mode_VentnActr16Mode_read)
  VentnActr16MovDir_VentnActr16MovDir/1 -> CCMVgaMotor_Runnable_sys/420(VentnActr16MovDir_VentnActr16MovDir_read)
  VentnActr16OvrTempErr_VentnActr16OvrTempErr/1 -> CCMVgaMotor_Runnable_sys/421(VentnActr16OvrTempErr_VentnActr16OvrTempErr_read)
  VentnActr16PsonSts_VentnActr16PsonSts/1 -> CCMVgaMotor_Runnable_sys/422(VentnActr16PsonSts_VentnActr16PsonSts_read)
  VentnActr16ResetSts_VentnActr16ResetSts/1 -> CCMVgaMotor_Runnable_sys/423(VentnActr16ResetSts_VentnActr16ResetSts_read)
  VentnActr16SpclFct_VentnActr16SpclFct/1 -> CCMVgaMotor_Runnable_sys/424(VentnActr16SpclFct_VentnActr16SpclFct_read)
  VentnActr16SpdSts_VentnActr16SpdSts/1 -> CCMVgaMotor_Runnable_sys/425(VentnActr16SpdSts_VentnActr16SpdSts_read)
  VentnActr16TrqSts_VentnActr16TrqSts/1 -> CCMVgaMotor_Runnable_sys/426(VentnActr16TrqSts_VentnActr16TrqSts_read)
  VentnActr16UrgcExist_VentnActr16UrgcExist/1 -> CCMVgaMotor_Runnable_sys/427(VentnActr16UrgcExist_VentnActr16UrgcExist_read)
  VentnActr16UrgcPson_VentnActr16UrgcPson/1 -> CCMVgaMotor_Runnable_sys/428(VentnActr16UrgcPson_VentnActr16UrgcPson_read)
  VentnActr16UrgcSts_VentnActr16UrgcSts/1 -> CCMVgaMotor_Runnable_sys/429(VentnActr16UrgcSts_VentnActr16UrgcSts_read)
  VentnActr16VoltErr_VentnActr16VoltErr/1 -> CCMVgaMotor_Runnable_sys/430(VentnActr16VoltErr_VentnActr16VoltErr_read)
  VentnActr17ActPson_VentnActr17ActPson/1 -> CCMVgaMotor_Runnable_sys/431(VentnActr17ActPson_VentnActr17ActPson_read)
  VentnActr17ActPson_VentnActr17ActPson_IsUpdated/1 -> CCMVgaMotor_Runnable_sys/432(VentnActr17ActPson_VentnActr17ActPson_IsUpdated_read)
  VentnActr17BlckInd_VentnActr17BlckInd/1 -> CCMVgaMotor_Runnable_sys/433(VentnActr17BlckInd_VentnActr17BlckInd_read)
  VentnActr17BlckSts_VentnActr17BlckSts/1 -> CCMVgaMotor_Runnable_sys/434(VentnActr17BlckSts_VentnActr17BlckSts_read)
  VentnActr17BusAdr_VentnActr17BusAdr/1 -> CCMVgaMotor_Runnable_sys/435(VentnActr17BusAdr_VentnActr17BusAdr_read)
  VentnActr17CoilSts_VentnActr17CoilSts/1 -> CCMVgaMotor_Runnable_sys/436(VentnActr17CoilSts_VentnActr17CoilSts_read)
  VentnActr17DrvDir_VentnActr17DrvDir/1 -> CCMVgaMotor_Runnable_sys/437(VentnActr17DrvDir_VentnActr17DrvDir_read)
  VentnActr17ElecErr_VentnActr17ElecErr/1 -> CCMVgaMotor_Runnable_sys/438(VentnActr17ElecErr_VentnActr17ElecErr_read)
  VentnActr17Mode_VentnActr17Mode/1 -> CCMVgaMotor_Runnable_sys/439(VentnActr17Mode_VentnActr17Mode_read)
  VentnActr17MovDir_VentnActr17MovDir/1 -> CCMVgaMotor_Runnable_sys/440(VentnActr17MovDir_VentnActr17MovDir_read)
  VentnActr17OvrTempErr_VentnActr17OvrTempErr/1 -> CCMVgaMotor_Runnable_sys/441(VentnActr17OvrTempErr_VentnActr17OvrTempErr_read)
  VentnActr17PsonSts_VentnActr17PsonSts/1 -> CCMVgaMotor_Runnable_sys/442(VentnActr17PsonSts_VentnActr17PsonSts_read)
  VentnActr17ResetSts_VentnActr17ResetSts/1 -> CCMVgaMotor_Runnable_sys/443(VentnActr17ResetSts_VentnActr17ResetSts_read)
  VentnActr17SpclFct_VentnActr17SpclFct/1 -> CCMVgaMotor_Runnable_sys/444(VentnActr17SpclFct_VentnActr17SpclFct_read)
  VentnActr17SpdSts_VentnActr17SpdSts/1 -> CCMVgaMotor_Runnable_sys/445(VentnActr17SpdSts_VentnActr17SpdSts_read)
  VentnActr17TrqSts_VentnActr17TrqSts/1 -> CCMVgaMotor_Runnable_sys/446(VentnActr17TrqSts_VentnActr17TrqSts_read)
  VentnActr17UrgcExist_VentnActr17UrgcExist/1 -> CCMVgaMotor_Runnable_sys/447(VentnActr17UrgcExist_VentnActr17UrgcExist_read)
  VentnActr17UrgcPson_VentnActr17UrgcPson/1 -> CCMVgaMotor_Runnable_sys/448(VentnActr17UrgcPson_VentnActr17UrgcPson_read)
  VentnActr17UrgcSts_VentnActr17UrgcSts/1 -> CCMVgaMotor_Runnable_sys/449(VentnActr17UrgcSts_VentnActr17UrgcSts_read)
  VentnActr17VoltErr_VentnActr17VoltErr/1 -> CCMVgaMotor_Runnable_sys/450(VentnActr17VoltErr_VentnActr17VoltErr_read)
  VentnActr18ActPson_VentnActr18ActPson/1 -> CCMVgaMotor_Runnable_sys/451(VentnActr18ActPson_VentnActr18ActPson_read)
  VentnActr18BlckInd_VentnActr18BlckInd/1 -> CCMVgaMotor_Runnable_sys/452(VentnActr18BlckInd_VentnActr18BlckInd_read)
  VentnActr18BlckSts_VentnActr18BlckSts/1 -> CCMVgaMotor_Runnable_sys/453(VentnActr18BlckSts_VentnActr18BlckSts_read)
  VentnActr18BusAdr_VentnActr18BusAdr/1 -> CCMVgaMotor_Runnable_sys/454(VentnActr18BusAdr_VentnActr18BusAdr_read)
  VentnActr18CoilSts_VentnActr18CoilSts/1 -> CCMVgaMotor_Runnable_sys/455(VentnActr18CoilSts_VentnActr18CoilSts_read)
  VentnActr18DrvDir_VentnActr18DrvDir/1 -> CCMVgaMotor_Runnable_sys/456(VentnActr18DrvDir_VentnActr18DrvDir_read)
  VentnActr18ElecErr_VentnActr18ElecErr/1 -> CCMVgaMotor_Runnable_sys/457(VentnActr18ElecErr_VentnActr18ElecErr_read)
  VentnActr18Mode_VentnActr18Mode/1 -> CCMVgaMotor_Runnable_sys/458(VentnActr18Mode_VentnActr18Mode_read)
  VentnActr18MovDir_VentnActr18MovDir/1 -> CCMVgaMotor_Runnable_sys/459(VentnActr18MovDir_VentnActr18MovDir_read)
  VentnActr18OvrTempErr_VentnActr18OvrTempErr/1 -> CCMVgaMotor_Runnable_sys/460(VentnActr18OvrTempErr_VentnActr18OvrTempErr_read)
  VentnActr18PsonSts_VentnActr18PsonSts/1 -> CCMVgaMotor_Runnable_sys/461(VentnActr18PsonSts_VentnActr18PsonSts_read)
  VentnActr18ResetSts_VentnActr18ResetSts/1 -> CCMVgaMotor_Runnable_sys/462(VentnActr18ResetSts_VentnActr18ResetSts_read)
  VentnActr18SpclFct_VentnActr18SpclFct/1 -> CCMVgaMotor_Runnable_sys/463(VentnActr18SpclFct_VentnActr18SpclFct_read)
  VentnActr18SpdSts_VentnActr18SpdSts/1 -> CCMVgaMotor_Runnable_sys/464(VentnActr18SpdSts_VentnActr18SpdSts_read)
  VentnActr18TrqSts_VentnActr18TrqSts/1 -> CCMVgaMotor_Runnable_sys/465(VentnActr18TrqSts_VentnActr18TrqSts_read)
  VentnActr18UrgcExist_VentnActr18UrgcExist/1 -> CCMVgaMotor_Runnable_sys/466(VentnActr18UrgcExist_VentnActr18UrgcExist_read)
  VentnActr18UrgcPson_VentnActr18UrgcPson/1 -> CCMVgaMotor_Runnable_sys/467(VentnActr18UrgcPson_VentnActr18UrgcPson_read)
  VentnActr18UrgcSts_VentnActr18UrgcSts/1 -> CCMVgaMotor_Runnable_sys/468(VentnActr18UrgcSts_VentnActr18UrgcSts_read)
  VentnActr18VoltErr_VentnActr18VoltErr/1 -> CCMVgaMotor_Runnable_sys/469(VentnActr18VoltErr_VentnActr18VoltErr_read)
  VentnActr19ActPson_VentnActr19ActPson/1 -> CCMVgaMotor_Runnable_sys/470(VentnActr19ActPson_VentnActr19ActPson_read)
  VentnActr19ActPson_VentnActr19ActPson_IsUpdated/1 -> CCMVgaMotor_Runnable_sys/471(VentnActr19ActPson_VentnActr19ActPson_IsUpdated_read)
  VentnActr19BlckInd_VentnActr19BlckInd/1 -> CCMVgaMotor_Runnable_sys/472(VentnActr19BlckInd_VentnActr19BlckInd_read)
  VentnActr19BlckSts_VentnActr19BlckSts/1 -> CCMVgaMotor_Runnable_sys/473(VentnActr19BlckSts_VentnActr19BlckSts_read)
  VentnActr19BusAdr_VentnActr19BusAdr/1 -> CCMVgaMotor_Runnable_sys/474(VentnActr19BusAdr_VentnActr19BusAdr_read)
  VentnActr19CoilSts_VentnActr19CoilSts/1 -> CCMVgaMotor_Runnable_sys/475(VentnActr19CoilSts_VentnActr19CoilSts_read)
  VentnActr19DrvDir_VentnActr19DrvDir/1 -> CCMVgaMotor_Runnable_sys/476(VentnActr19DrvDir_VentnActr19DrvDir_read)
  VentnActr19ElecErr_VentnActr19ElecErr/1 -> CCMVgaMotor_Runnable_sys/477(VentnActr19ElecErr_VentnActr19ElecErr_read)
  VentnActr19Mode_VentnActr19Mode/1 -> CCMVgaMotor_Runnable_sys/478(VentnActr19Mode_VentnActr19Mode_read)
  VentnActr19MovDir_VentnActr19MovDir/1 -> CCMVgaMotor_Runnable_sys/479(VentnActr19MovDir_VentnActr19MovDir_read)
  VentnActr19OvrTempErr_VentnActr19OvrTempErr/1 -> CCMVgaMotor_Runnable_sys/480(VentnActr19OvrTempErr_VentnActr19OvrTempErr_read)
  VentnActr19PsonSts_VentnActr19PsonSts/1 -> CCMVgaMotor_Runnable_sys/481(VentnActr19PsonSts_VentnActr19PsonSts_read)
  VentnActr19ResetSts_VentnActr19ResetSts/1 -> CCMVgaMotor_Runnable_sys/482(VentnActr19ResetSts_VentnActr19ResetSts_read)
  VentnActr19SpclFct_VentnActr19SpclFct/1 -> CCMVgaMotor_Runnable_sys/483(VentnActr19SpclFct_VentnActr19SpclFct_read)
  VentnActr19SpdSts_VentnActr19SpdSts/1 -> CCMVgaMotor_Runnable_sys/484(VentnActr19SpdSts_VentnActr19SpdSts_read)
  VentnActr19TrqSts_VentnActr19TrqSts/1 -> CCMVgaMotor_Runnable_sys/485(VentnActr19TrqSts_VentnActr19TrqSts_read)
  VentnActr19UrgcExist_VentnActr19UrgcExist/1 -> CCMVgaMotor_Runnable_sys/486(VentnActr19UrgcExist_VentnActr19UrgcExist_read)
  VentnActr19UrgcPson_VentnActr19UrgcPson/1 -> CCMVgaMotor_Runnable_sys/487(VentnActr19UrgcPson_VentnActr19UrgcPson_read)
  VentnActr19UrgcSts_VentnActr19UrgcSts/1 -> CCMVgaMotor_Runnable_sys/488(VentnActr19UrgcSts_VentnActr19UrgcSts_read)
  VentnActr19VoltErr_VentnActr19VoltErr/1 -> CCMVgaMotor_Runnable_sys/489(VentnActr19VoltErr_VentnActr19VoltErr_read)
  VentnActr20ActPson_VentnActr20ActPson/1 -> CCMVgaMotor_Runnable_sys/490(VentnActr20ActPson_VentnActr20ActPson_read)
  VentnActr20ActPson_VentnActr20ActPson_IsUpdated/1 -> CCMVgaMotor_Runnable_sys/491(VentnActr20ActPson_VentnActr20ActPson_IsUpdated_read)
  VentnActr20BlckInd_VentnActr20BlckInd/1 -> CCMVgaMotor_Runnable_sys/492(VentnActr20BlckInd_VentnActr20BlckInd_read)
  VentnActr20BlckSts_VentnActr20BlckSts/1 -> CCMVgaMotor_Runnable_sys/493(VentnActr20BlckSts_VentnActr20BlckSts_read)
  VentnActr20BusAdr_VentnActr20BusAdr/1 -> CCMVgaMotor_Runnable_sys/494(VentnActr20BusAdr_VentnActr20BusAdr_read)
  VentnActr20CoilSts_VentnActr20CoilSts/1 -> CCMVgaMotor_Runnable_sys/495(VentnActr20CoilSts_VentnActr20CoilSts_read)
  VentnActr20DrvDir_VentnActr20DrvDir/1 -> CCMVgaMotor_Runnable_sys/496(VentnActr20DrvDir_VentnActr20DrvDir_read)
  VentnActr20ElecErr_VentnActr20ElecErr/1 -> CCMVgaMotor_Runnable_sys/497(VentnActr20ElecErr_VentnActr20ElecErr_read)
  VentnActr20Mode_VentnActr20Mode/1 -> CCMVgaMotor_Runnable_sys/498(VentnActr20Mode_VentnActr20Mode_read)
  VentnActr20MovDir_VentnActr20MovDir/1 -> CCMVgaMotor_Runnable_sys/499(VentnActr20MovDir_VentnActr20MovDir_read)
  VentnActr20OvrTempErr_VentnActr20OvrTempErr/1 -> CCMVgaMotor_Runnable_sys/500(VentnActr20OvrTempErr_VentnActr20OvrTempErr_read)
  VentnActr20PsonSts_VentnActr20PsonSts/1 -> CCMVgaMotor_Runnable_sys/501(VentnActr20PsonSts_VentnActr20PsonSts_read)
  VentnActr20ResetSts_VentnActr20ResetSts/1 -> CCMVgaMotor_Runnable_sys/502(VentnActr20ResetSts_VentnActr20ResetSts_read)
  VentnActr20SpclFct_VentnActr20SpclFct/1 -> CCMVgaMotor_Runnable_sys/503(VentnActr20SpclFct_VentnActr20SpclFct_read)
  VentnActr20SpdSts_VentnActr20SpdSts/1 -> CCMVgaMotor_Runnable_sys/504(VentnActr20SpdSts_VentnActr20SpdSts_read)
  VentnActr20TrqSts_VentnActr20TrqSts/1 -> CCMVgaMotor_Runnable_sys/505(VentnActr20TrqSts_VentnActr20TrqSts_read)
  VentnActr20UrgcExist_VentnActr20UrgcExist/1 -> CCMVgaMotor_Runnable_sys/506(VentnActr20UrgcExist_VentnActr20UrgcExist_read)
  VentnActr20UrgcPson_VentnActr20UrgcPson/1 -> CCMVgaMotor_Runnable_sys/507(VentnActr20UrgcPson_VentnActr20UrgcPson_read)
  VentnActr20UrgcSts_VentnActr20UrgcSts/1 -> CCMVgaMotor_Runnable_sys/508(VentnActr20UrgcSts_VentnActr20UrgcSts_read)
  VentnActr20VoltErr_VentnActr20VoltErr/1 -> CCMVgaMotor_Runnable_sys/509(VentnActr20VoltErr_VentnActr20VoltErr_read)
  VGAStopDuePowerLimit_VGAStopDuePowerLimit/1 -> CCMVgaMotor_Runnable_sys/510(VGAStopDuePowerLimit_VGAStopDuePowerLimit_read)
  CCMVgaMotor_Runnable_sys/1(VentnMotAdr_VentnMotAdr_write) -> VentnMotAdr_VentnMotAdr/1
  CCMVgaMotor_Runnable_sys/2(VentnMotAdr2_VentnMotAdr2_write) -> VentnMotAdr2_VentnMotAdr2/1
  CCMVgaMotor_Runnable_sys/3(VentnMotBlockCmd_VentnMotBlockCmd_write) -> VentnMotBlockCmd_VentnMotBlockCmd/1
  CCMVgaMotor_Runnable_sys/4(VentnMotBlockCmd2_VentnMotBlockCmd2_write) -> VentnMotBlockCmd2_VentnMotBlockCmd2/1
  CCMVgaMotor_Runnable_sys/5(VentnMotClearReq_VentnMotClearReq_write) -> VentnMotClearReq_VentnMotClearReq/1
  CCMVgaMotor_Runnable_sys/6(VentnMotClearReq2_VentnMotClearReq2_write) -> VentnMotClearReq2_VentnMotClearReq2/1
  CCMVgaMotor_Runnable_sys/7(VentnMotCoilPwrOn_VentnMotCoilPwrOn_write) -> VentnMotCoilPwrOn_VentnMotCoilPwrOn/1
  CCMVgaMotor_Runnable_sys/8(VentnMotCoilPwrOn2_VentnMotCoilPwrOn2_write) -> VentnMotCoilPwrOn2_VentnMotCoilPwrOn2/1
  CCMVgaMotor_Runnable_sys/9(VentnMotDir_VentnMotDir_write) -> VentnMotDir_VentnMotDir/1
  CCMVgaMotor_Runnable_sys/10(VentnMotDir2_VentnMotDir2_write) -> VentnMotDir2_VentnMotDir2/1
  CCMVgaMotor_Runnable_sys/11(VentnMotMode_VentnMotMode_write) -> VentnMotMode_VentnMotMode/1
  CCMVgaMotor_Runnable_sys/12(VentnMotMode2_VentnMotMode2_write) -> VentnMotMode2_VentnMotMode2/1
  CCMVgaMotor_Runnable_sys/13(VentnMotPsonAvl_VentnMotPsonAvl_write) -> VentnMotPsonAvl_VentnMotPsonAvl/1
  CCMVgaMotor_Runnable_sys/14(VentnMotPsonAvl2_VentnMotPsonAvl2_write) -> VentnMotPsonAvl2_VentnMotPsonAvl2/1
  CCMVgaMotor_Runnable_sys/15(VentnMotSaveData_VentnMotSaveData_write) -> VentnMotSaveData_VentnMotSaveData/1
  CCMVgaMotor_Runnable_sys/16(VentnMotSaveData2_VentnMotSaveData2_write) -> VentnMotSaveData2_VentnMotSaveData2/1
  CCMVgaMotor_Runnable_sys/17(VentnMotSpdSet_VentnMotSpdSet_write) -> VentnMotSpdSet_VentnMotSpdSet/1
  CCMVgaMotor_Runnable_sys/18(VentnMotSpdSet2_VentnMotSpdSet2_write) -> VentnMotSpdSet2_VentnMotSpdSet2/1
  CCMVgaMotor_Runnable_sys/19(VentnMotStrtPson_VentnMotStrtPson_write) -> VentnMotStrtPson_VentnMotStrtPson/1
  CCMVgaMotor_Runnable_sys/20(VentnMotStrtPson2_VentnMotStrtPson2_write) -> VentnMotStrtPson2_VentnMotStrtPson2/1
  CCMVgaMotor_Runnable_sys/21(VentnMotTargtPson_VentnMotTargtPson_write) -> VentnMotTargtPson_VentnMotTargtPson/1
  CCMVgaMotor_Runnable_sys/22(VentnMotTargtPson2_VentnMotTargtPson2_write) -> VentnMotTargtPson2_VentnMotTargtPson2/1
  CCMVgaMotor_Runnable_sys/23(VentnMotUrgcPson_VentnMotUrgcPson_write) -> VentnMotUrgcPson_VentnMotUrgcPson/1
  CCMVgaMotor_Runnable_sys/24(VentnMotUrgcPson2_VentnMotUrgcPson2_write) -> VentnMotUrgcPson2_VentnMotUrgcPson2/1
  CCMVgaMotor_Runnable_sys/25(VentnMotUrgcUnlck_VentnMotUrgcUnlck_write) -> VentnMotUrgcUnlck_VentnMotUrgcUnlck/1
  CCMVgaMotor_Runnable_sys/26(VentnMotUrgcUnlck2_VentnMotUrgcUnlck2_write) -> VentnMotUrgcUnlck2_VentnMotUrgcUnlck2/1
  BswLifeCycle_LifeCycle/1 -> CCMVgaMotor_Runnable_sys/511(BswLifeCycle_LifeCycle_read)
  SbcPwrOn_SbcPwrOn/1 -> [SbcPwrOn_SbcPwrOn_Tag]
  [SbcPwrOn_SbcPwrOn_Tag] -> CCMVgaMotor_Init/2(SbcPwrOn_SbcPwrOn_read)
  [SbcPwrOn_SbcPwrOn_Tag] -> CCMVgaMotor_Runnable_sys/103(SbcPwrOn_SbcPwrOn_read)
  SbcWakeupSta_SbcWakeupSta/1 -> [SbcWakeupSta_SbcWakeupSta_Tag]
  [SbcWakeupSta_SbcWakeupSta_Tag] -> CCMVgaMotor_Init/1(SbcWakeupSta_SbcWakeupSta_read)
  [SbcWakeupSta_SbcWakeupSta_Tag] -> CCMVgaMotor_Runnable_sys/104(SbcWakeupSta_SbcWakeupSta_read)
  CCMVgaMotor_50msRunnable/1 -> CCMVgaMotor_50msRunnable_sys/Trigger
  CCMVgaMotor_Runnable/1 -> CCMVgaMotor_Runnable_sys/Trigger
  CCMVGAMotor_VentnMotAdr/1 -> CCMVGAMotor_VentnMotAdr_sys/Trigger
  CCMVGAMotor_VentnMotAdr2/1 -> CCMVGAMotor_VentnMotAdr2_sys/Trigger
